simulink model "os_polyphase_synth_4096c"  (R2021a) (continued)
== system os_polyphase_synth_4096c/pfb_fir/pol1_in1_tap3 == (continued)
      | P-(P+CARRYIN)
      | P-(P+CONCAT)
      | P-(P+CONCAT+C)
      | P-(P+CONCAT+C+CARRYIN)
      | P-(P+CONCAT+CARRYIN)
      | P-(P+D)
      | P-(P+D*B)
      | P-(P+D*B+CARRYIN)
      | P-(P+D*BCIN)
      | P-(P+D*BCIN+CARRYIN)
      | P-(P+D*D)
      | P-(P+D*D+CARRYIN)
      | P-(P+D+CARRYIN)
      | P-(P+P)
      | P-(P+P+C)
      | P-(P+P+C+CARRYIN)
      | P-(P+P+CARRYIN)
      | P-A
      | P-A*A
      | P-A*A-C
      | P-A*A-C-CARRYIN
      | P-A*A-CARRYIN
      | P-A*A-P
      | P-A*A-P-CARRYIN
      | P-A*B
      | P-A*B-C
      | P-A*B-C-CARRYIN
      | P-A*B-CARRYIN
      | P-A*B-P
      | P-A*B-P-CARRYIN
      | P-A*BCIN
      | P-A*BCIN-C
      | P-A*BCIN-C-CARRYIN
      | P-A*BCIN-CARRYIN
      | P-A*BCIN-P
      | P-A*BCIN-P-CARRYIN
      | P-A-C
      | P-A-C-CARRYIN
      | P-A-CARRYIN
      | P-A-P
      | P-A-P-CARRYIN
      | P-ACIN
      | P-ACIN*ACIN
      | P-ACIN*ACIN-C
      | P-ACIN*ACIN-C-CARRYIN
      | P-ACIN*ACIN-CARRYIN
      | P-ACIN*ACIN-P
      | P-ACIN*ACIN-P-CARRYIN
      | P-ACIN*B
      | P-ACIN*B-C
      | P-ACIN*B-C-CARRYIN
      | P-ACIN*B-CARRYIN
      | P-ACIN*B-P
      | P-ACIN*B-P-CARRYIN
      | P-ACIN*BCIN
      | P-ACIN*BCIN-C
      | P-ACIN*BCIN-C-CARRYIN
      | P-ACIN*BCIN-CARRYIN
      | P-ACIN*BCIN-P
      | P-ACIN*BCIN-P-CARRYIN
      | P-ACIN-C
      | P-ACIN-C-CARRYIN
      | P-ACIN-CARRYIN
      | P-ACIN-P
      | P-ACIN-P-CARRYIN
      | P-B*(A+D)
      | P-B*(A+D)-C
      | P-B*(A+D)-C-CARRYIN
      | P-B*(A+D)-CARRYIN
      | P-B*(A+D)-P
      | P-B*(A+D)-P-CARRYIN
      | P-B*(ACIN+D)
      | P-B*(ACIN+D)-C
      | P-B*(ACIN+D)-C-CARRYIN
      | P-B*(ACIN+D)-CARRYIN
      | P-B*(ACIN+D)-P
      | P-B*(ACIN+D)-P-CARRYIN
      | P-B*(D+A)
      | P-B*(D+A)-C
      | P-B*(D+A)-C-CARRYIN
      | P-B*(D+A)-CARRYIN
      | P-B*(D+A)-P
      | P-B*(D+A)-P-CARRYIN
      | P-B*(D+ACIN)
      | P-B*(D+ACIN)-C
      | P-B*(D+ACIN)-C-CARRYIN
      | P-B*(D+ACIN)-CARRYIN
      | P-B*(D+ACIN)-P
      | P-B*(D+ACIN)-P-CARRYIN
      | P-B*(D-A)
      | P-B*(D-A)-C
      | P-B*(D-A)-C-CARRYIN
      | P-B*(D-A)-CARRYIN
      | P-B*(D-A)-P
      | P-B*(D-A)-P-CARRYIN
      | P-B*(D-ACIN)
      | P-B*(D-ACIN)-C
      | P-B*(D-ACIN)-C-CARRYIN
      | P-B*(D-ACIN)-CARRYIN
      | P-B*(D-ACIN)-P
      | P-B*(D-ACIN)-P-CARRYIN
      | P-B*A
      | P-B*A-C
      | P-B*A-C-CARRYIN
      | P-B*A-CARRYIN
      | P-B*A-P
      | P-B*A-P-CARRYIN
      | P-B*ACIN
      | P-B*ACIN-C
      | P-B*ACIN-C-CARRYIN
      | P-B*ACIN-CARRYIN
      | P-B*ACIN-P
      | P-B*ACIN-P-CARRYIN
      | P-B*D
      | P-B*D-C
      | P-B*D-C-CARRYIN
      | P-B*D-CARRYIN
      | P-B*D-P
      | P-B*D-P-CARRYIN
      | P-BCIN*(A+D)
      | P-BCIN*(A+D)-C
      | P-BCIN*(A+D)-C-CARRYIN
      | P-BCIN*(A+D)-CARRYIN
      | P-BCIN*(A+D)-P
      | P-BCIN*(A+D)-P-CARRYIN
      | P-BCIN*(ACIN+D)
      | P-BCIN*(ACIN+D)-C
      | P-BCIN*(ACIN+D)-C-CARRYIN
      | P-BCIN*(ACIN+D)-CARRYIN
      | P-BCIN*(ACIN+D)-P
      | P-BCIN*(ACIN+D)-P-CARRYIN
      | P-BCIN*(D+A)
      | P-BCIN*(D+A)-C
      | P-BCIN*(D+A)-C-CARRYIN
      | P-BCIN*(D+A)-CARRYIN
      | P-BCIN*(D+A)-P
      | P-BCIN*(D+A)-P-CARRYIN
      | P-BCIN*(D+ACIN)
      | P-BCIN*(D+ACIN)-C
      | P-BCIN*(D+ACIN)-C-CARRYIN
      | P-BCIN*(D+ACIN)-CARRYIN
      | P-BCIN*(D+ACIN)-P
      | P-BCIN*(D+ACIN)-P-CARRYIN
      | P-BCIN*(D-A)
      | P-BCIN*(D-A)-C
      | P-BCIN*(D-A)-C-CARRYIN
      | P-BCIN*(D-A)-CARRYIN
      | P-BCIN*(D-A)-P
      | P-BCIN*(D-A)-P-CARRYIN
      | P-BCIN*(D-ACIN)
      | P-BCIN*(D-ACIN)-C
      | P-BCIN*(D-ACIN)-C-CARRYIN
      | P-BCIN*(D-ACIN)-CARRYIN
      | P-BCIN*(D-ACIN)-P
      | P-BCIN*(D-ACIN)-P-CARRYIN
      | P-BCIN*A
      | P-BCIN*A-C
      | P-BCIN*A-C-CARRYIN
      | P-BCIN*A-CARRYIN
      | P-BCIN*A-P
      | P-BCIN*A-P-CARRYIN
      | P-BCIN*ACIN
      | P-BCIN*ACIN-C
      | P-BCIN*ACIN-C-CARRYIN
      | P-BCIN*ACIN-CARRYIN
      | P-BCIN*ACIN-P
      | P-BCIN*ACIN-P-CARRYIN
      | P-BCIN*D
      | P-BCIN*D-C
      | P-BCIN*D-C-CARRYIN
      | P-BCIN*D-CARRYIN
      | P-BCIN*D-P
      | P-BCIN*D-P-CARRYIN
      | P-C
      | P-C-(A+D)
      | P-C-(A+D)*(A+D)
      | P-C-(A+D)*(A+D)-CARRYIN
      | P-C-(A+D)*B
      | P-C-(A+D)*B-CARRYIN
      | P-C-(A+D)*BCIN
      | P-C-(A+D)*BCIN-CARRYIN
      | P-C-(A+D)-CARRYIN
      | P-C-(ACIN+D)
      | P-C-(ACIN+D)*(ACIN+D)
      | P-C-(ACIN+D)*(ACIN+D)-CARRYIN
      | P-C-(ACIN+D)*B
      | P-C-(ACIN+D)*B-CARRYIN
      | P-C-(ACIN+D)*BCIN
      | P-C-(ACIN+D)*BCIN-CARRYIN
      | P-C-(ACIN+D)-CARRYIN
      | P-C-(D+A)
      | P-C-(D+A)*(D+A)
      | P-C-(D+A)*(D+A)-CARRYIN
      | P-C-(D+A)*B
      | P-C-(D+A)*B-CARRYIN
      | P-C-(D+A)*BCIN
      | P-C-(D+A)*BCIN-CARRYIN
      | P-C-(D+A)-CARRYIN
      | P-C-(D+ACIN)
      | P-C-(D+ACIN)*(D+ACIN)
      | P-C-(D+ACIN)*(D+ACIN)-CARRYIN
      | P-C-(D+ACIN)*B
      | P-C-(D+ACIN)*B-CARRYIN
      | P-C-(D+ACIN)*BCIN
      | P-C-(D+ACIN)*BCIN-CARRYIN
      | P-C-(D+ACIN)-CARRYIN
      | P-C-(D-A)
      | P-C-(D-A)*(D-A)
      | P-C-(D-A)*(D-A)-CARRYIN
      | P-C-(D-A)*B
      | P-C-(D-A)*B-CARRYIN
      | P-C-(D-A)*BCIN
      | P-C-(D-A)*BCIN-CARRYIN
      | P-C-(D-A)-CARRYIN
      | P-C-(D-ACIN)
      | P-C-(D-ACIN)*(D-ACIN)
      | P-C-(D-ACIN)*(D-ACIN)-CARRYIN
      | P-C-(D-ACIN)*B
      | P-C-(D-ACIN)*B-CARRYIN
      | P-C-(D-ACIN)*BCIN
      | P-C-(D-ACIN)*BCIN-CARRYIN
      | P-C-(D-ACIN)-CARRYIN
      | P-C-A
      | P-C-A*A
      | P-C-A*A-CARRYIN
      | P-C-A*B
      | P-C-A*B-CARRYIN
      | P-C-A*BCIN
      | P-C-A*BCIN-CARRYIN
      | P-C-A-CARRYIN
      | P-C-ACIN
      | P-C-ACIN*ACIN
      | P-C-ACIN*ACIN-CARRYIN
      | P-C-ACIN*B
      | P-C-ACIN*B-CARRYIN
      | P-C-ACIN*BCIN
      | P-C-ACIN*BCIN-CARRYIN
      | P-C-ACIN-CARRYIN
      | P-C-B*(A+D)
      | P-C-B*(A+D)-CARRYIN
      | P-C-B*(ACIN+D)
      | P-C-B*(ACIN+D)-CARRYIN
      | P-C-B*(D+A)
      | P-C-B*(D+A)-CARRYIN
      | P-C-B*(D+ACIN)
      | P-C-B*(D+ACIN)-CARRYIN
      | P-C-B*(D-A)
      | P-C-B*(D-A)-CARRYIN
      | P-C-B*(D-ACIN)
      | P-C-B*(D-ACIN)-CARRYIN
      | P-C-B*A
      | P-C-B*A-CARRYIN
      | P-C-B*ACIN
      | P-C-B*ACIN-CARRYIN
      | P-C-B*D
      | P-C-B*D-CARRYIN
      | P-C-BCIN*(A+D)
      | P-C-BCIN*(A+D)-CARRYIN
      | P-C-BCIN*(ACIN+D)
      | P-C-BCIN*(ACIN+D)-CARRYIN
      | P-C-BCIN*(D+A)
      | P-C-BCIN*(D+A)-CARRYIN
      | P-C-BCIN*(D+ACIN)
      | P-C-BCIN*(D+ACIN)-CARRYIN
      | P-C-BCIN*(D-A)
      | P-C-BCIN*(D-A)-CARRYIN
      | P-C-BCIN*(D-ACIN)
      | P-C-BCIN*(D-ACIN)-CARRYIN
      | P-C-BCIN*A
      | P-C-BCIN*A-CARRYIN
      | P-C-BCIN*ACIN
      | P-C-BCIN*ACIN-CARRYIN
      | P-C-BCIN*D
      | P-C-BCIN*D-CARRYIN
      | P-C-C
      | P-C-C-CARRYIN
      | P-C-C-CONCAT
      | P-C-C-CONCAT-CARRYIN
      | P-C-C-P
      | P-C-C-P-CARRYIN
      | P-C-CARRYCASCIN
      | P-C-CARRYIN
      | P-C-CONCAT
      | P-C-CONCAT-C
      | P-C-CONCAT-C-CARRYIN
      | P-C-CONCAT-CARRYIN
      | P-C-CONCAT-P
      | P-C-CONCAT-P-CARRYIN
      | P-C-D
      | P-C-D*B
      | P-C-D*B-CARRYIN
      | P-C-D*BCIN
      | P-C-D*BCIN-CARRYIN
      | P-C-D*D
      | P-C-D*D-CARRYIN
      | P-C-D-CARRYIN
      | P-C-P
      | P-C-P-C
      | P-C-P-C-CARRYIN
      | P-C-P-CARRYIN
      | P-C-P-CONCAT
      | P-C-P-CONCAT-CARRYIN
      | P-C-P-P
      | P-C-P-P-CARRYIN
      | P-CARRYCASCIN
      | P-CARRYIN
      | P-CONCAT
      | P-CONCAT-C
      | P-CONCAT-C-C
      | P-CONCAT-C-C-CARRYIN
      | P-CONCAT-C-CARRYIN
      | P-CONCAT-C-P
      | P-CONCAT-C-P-CARRYIN
      | P-CONCAT-CARRYCASCIN
      | P-CONCAT-CARRYIN
      | P-CONCAT-P
      | P-CONCAT-P-C
      | P-CONCAT-P-C-CARRYIN
      | P-CONCAT-P-CARRYIN
      | P-D
      | P-D*B
      | P-D*B-C
      | P-D*B-C-CARRYIN
      | P-D*B-CARRYIN
      | P-D*B-P
      | P-D*B-P-CARRYIN
      | P-D*BCIN
      | P-D*BCIN-C
      | P-D*BCIN-C-CARRYIN
      | P-D*BCIN-CARRYIN
      | P-D*BCIN-P
      | P-D*BCIN-P-CARRYIN
      | P-D*D
      | P-D*D-C
      | P-D*D-C-CARRYIN
      | P-D*D-CARRYIN
      | P-D*D-P
      | P-D*D-P-CARRYIN
      | P-D-C
      | P-D-C-CARRYIN
      | P-D-CARRYIN
      | P-D-P
      | P-D-P-CARRYIN
      | P-P
      | P-P-(A+D)
      | P-P-(A+D)*(A+D)
      | P-P-(A+D)*(A+D)-CARRYIN
      | P-P-(A+D)*B
      | P-P-(A+D)*B-CARRYIN
      | P-P-(A+D)*BCIN
      | P-P-(A+D)*BCIN-CARRYIN
      | P-P-(A+D)-CARRYIN
      | P-P-(ACIN+D)
      | P-P-(ACIN+D)*(ACIN+D)
      | P-P-(ACIN+D)*(ACIN+D)-CARRYIN
      | P-P-(ACIN+D)*B
      | P-P-(ACIN+D)*B-CARRYIN
      | P-P-(ACIN+D)*BCIN
      | P-P-(ACIN+D)*BCIN-CARRYIN
      | P-P-(ACIN+D)-CARRYIN
      | P-P-(D+A)
      | P-P-(D+A)*(D+A)
      | P-P-(D+A)*(D+A)-CARRYIN
      | P-P-(D+A)*B
      | P-P-(D+A)*B-CARRYIN
      | P-P-(D+A)*BCIN
      | P-P-(D+A)*BCIN-CARRYIN
      | P-P-(D+A)-CARRYIN
      | P-P-(D+ACIN)
      | P-P-(D+ACIN)*(D+ACIN)
      | P-P-(D+ACIN)*(D+ACIN)-CARRYIN
      | P-P-(D+ACIN)*B
      | P-P-(D+ACIN)*B-CARRYIN
      | P-P-(D+ACIN)*BCIN
      | P-P-(D+ACIN)*BCIN-CARRYIN
      | P-P-(D+ACIN)-CARRYIN
      | P-P-(D-A)
      | P-P-(D-A)*(D-A)
      | P-P-(D-A)*(D-A)-CARRYIN
      | P-P-(D-A)*B
      | P-P-(D-A)*B-CARRYIN
      | P-P-(D-A)*BCIN
      | P-P-(D-A)*BCIN-CARRYIN
      | P-P-(D-A)-CARRYIN
      | P-P-(D-ACIN)
      | P-P-(D-ACIN)*(D-ACIN)
      | P-P-(D-ACIN)*(D-ACIN)-CARRYIN
      | P-P-(D-ACIN)*B
      | P-P-(D-ACIN)*B-CARRYIN
      | P-P-(D-ACIN)*BCIN
      | P-P-(D-ACIN)*BCIN-CARRYIN
      | P-P-(D-ACIN)-CARRYIN
      | P-P-A
      | P-P-A*A
      | P-P-A*A-CARRYIN
      | P-P-A*B
      | P-P-A*B-CARRYIN
      | P-P-A*BCIN
      | P-P-A*BCIN-CARRYIN
      | P-P-A-CARRYIN
      | P-P-ACIN
      | P-P-ACIN*ACIN
      | P-P-ACIN*ACIN-CARRYIN
      | P-P-ACIN*B
      | P-P-ACIN*B-CARRYIN
      | P-P-ACIN*BCIN
      | P-P-ACIN*BCIN-CARRYIN
      | P-P-ACIN-CARRYIN
      | P-P-B*(A+D)
      | P-P-B*(A+D)-CARRYIN
      | P-P-B*(ACIN+D)
      | P-P-B*(ACIN+D)-CARRYIN
      | P-P-B*(D+A)
      | P-P-B*(D+A)-CARRYIN
      | P-P-B*(D+ACIN)
      | P-P-B*(D+ACIN)-CARRYIN
      | P-P-B*(D-A)
      | P-P-B*(D-A)-CARRYIN
      | P-P-B*(D-ACIN)
      | P-P-B*(D-ACIN)-CARRYIN
      | P-P-B*A
      | P-P-B*A-CARRYIN
      | P-P-B*ACIN
      | P-P-B*ACIN-CARRYIN
      | P-P-B*D
      | P-P-B*D-CARRYIN
      | P-P-BCIN*(A+D)
      | P-P-BCIN*(A+D)-CARRYIN
      | P-P-BCIN*(ACIN+D)
      | P-P-BCIN*(ACIN+D)-CARRYIN
      | P-P-BCIN*(D+A)
      | P-P-BCIN*(D+A)-CARRYIN
      | P-P-BCIN*(D+ACIN)
      | P-P-BCIN*(D+ACIN)-CARRYIN
      | P-P-BCIN*(D-A)
      | P-P-BCIN*(D-A)-CARRYIN
      | P-P-BCIN*(D-ACIN)
      | P-P-BCIN*(D-ACIN)-CARRYIN
      | P-P-BCIN*A
      | P-P-BCIN*A-CARRYIN
      | P-P-BCIN*ACIN
      | P-P-BCIN*ACIN-CARRYIN
      | P-P-BCIN*D
      | P-P-BCIN*D-CARRYIN
      | P-P-C
      | P-P-C-C
      | P-P-C-C-CARRYIN
      | P-P-C-CARRYIN
      | P-P-C-CONCAT
      | P-P-C-CONCAT-CARRYIN
      | P-P-C-P
      | P-P-C-P-CARRYIN
      | P-P-CARRYCASCIN
      | P-P-CARRYIN
      | P-P-CONCAT
      | P-P-CONCAT-C
      | P-P-CONCAT-C-CARRYIN
      | P-P-CONCAT-CARRYIN
      | P-P-D
      | P-P-D*B
      | P-P-D*B-CARRYIN
      | P-P-D*BCIN
      | P-P-D*BCIN-CARRYIN
      | P-P-D*D
      | P-P-D*D-CARRYIN
      | P-P-D-CARRYIN
      | P-P-P
      | P-P-P-C
      | P-P-P-C-CARRYIN
      | P-P-P-CARRYIN
      | P-P>>17
      | P-PCIN
      | P-PCIN>>17
      | P>>17
      | P>>17+(A+D)
      | P>>17+(A+D)*(A+D)
      | P>>17+(A+D)*(A+D)+C
      | P>>17+(A+D)*(A+D)+C+CARRYIN
      | P>>17+(A+D)*(A+D)+CARRYIN
      | P>>17+(A+D)*(A+D)+P
      | P>>17+(A+D)*(A+D)+P+CARRYIN
      | P>>17+(A+D)*B
      | P>>17+(A+D)*B+C
      | P>>17+(A+D)*B+C+CARRYIN
      | P>>17+(A+D)*B+CARRYIN
      | P>>17+(A+D)*B+P
      | P>>17+(A+D)*B+P+CARRYIN
      | P>>17+(A+D)*BCIN
      | P>>17+(A+D)*BCIN+C
      | P>>17+(A+D)*BCIN+C+CARRYIN
      | P>>17+(A+D)*BCIN+CARRYIN
      | P>>17+(A+D)*BCIN+P
      | P>>17+(A+D)*BCIN+P+CARRYIN
      | P>>17+(A+D)+C
      | P>>17+(A+D)+C+CARRYIN
      | P>>17+(A+D)+CARRYIN
      | P>>17+(A+D)+P
      | P>>17+(A+D)+P+CARRYIN
      | P>>17+(ACIN+D)
      | P>>17+(ACIN+D)*(ACIN+D)
      | P>>17+(ACIN+D)*(ACIN+D)+C
      | P>>17+(ACIN+D)*(ACIN+D)+C+CARRYIN
      | P>>17+(ACIN+D)*(ACIN+D)+CARRYIN
      | P>>17+(ACIN+D)*(ACIN+D)+P
      | P>>17+(ACIN+D)*(ACIN+D)+P+CARRYIN
      | P>>17+(ACIN+D)*B
      | P>>17+(ACIN+D)*B+C
      | P>>17+(ACIN+D)*B+C+CARRYIN
      | P>>17+(ACIN+D)*B+CARRYIN
      | P>>17+(ACIN+D)*B+P
      | P>>17+(ACIN+D)*B+P+CARRYIN
      | P>>17+(ACIN+D)*BCIN
      | P>>17+(ACIN+D)*BCIN+C
      | P>>17+(ACIN+D)*BCIN+C+CARRYIN
      | P>>17+(ACIN+D)*BCIN+CARRYIN
      | P>>17+(ACIN+D)*BCIN+P
      | P>>17+(ACIN+D)*BCIN+P+CARRYIN
      | P>>17+(ACIN+D)+C
      | P>>17+(ACIN+D)+C+CARRYIN
      | P>>17+(ACIN+D)+CARRYIN
      | P>>17+(ACIN+D)+P
      | P>>17+(ACIN+D)+P+CARRYIN
      | P>>17+(D+A)
      | P>>17+(D+A)*(D+A)
      | P>>17+(D+A)*(D+A)+C
      | P>>17+(D+A)*(D+A)+C+CARRYIN
      | P>>17+(D+A)*(D+A)+CARRYIN
      | P>>17+(D+A)*(D+A)+P
      | P>>17+(D+A)*(D+A)+P+CARRYIN
      | P>>17+(D+A)*B
      | P>>17+(D+A)*B+C
      | P>>17+(D+A)*B+C+CARRYIN
      | P>>17+(D+A)*B+CARRYIN
      | P>>17+(D+A)*B+P
      | P>>17+(D+A)*B+P+CARRYIN
      | P>>17+(D+A)*BCIN
      | P>>17+(D+A)*BCIN+C
      | P>>17+(D+A)*BCIN+C+CARRYIN
      | P>>17+(D+A)*BCIN+CARRYIN
      | P>>17+(D+A)*BCIN+P
      | P>>17+(D+A)*BCIN+P+CARRYIN
      | P>>17+(D+A)+C
      | P>>17+(D+A)+C+CARRYIN
      | P>>17+(D+A)+CARRYIN
      | P>>17+(D+A)+P
      | P>>17+(D+A)+P+CARRYIN
      | P>>17+(D+ACIN)
      | P>>17+(D+ACIN)*(D+ACIN)
      | P>>17+(D+ACIN)*(D+ACIN)+C
      | P>>17+(D+ACIN)*(D+ACIN)+C+CARRYIN
      | P>>17+(D+ACIN)*(D+ACIN)+CARRYIN
      | P>>17+(D+ACIN)*(D+ACIN)+P
      | P>>17+(D+ACIN)*(D+ACIN)+P+CARRYIN
      | P>>17+(D+ACIN)*B
      | P>>17+(D+ACIN)*B+C
      | P>>17+(D+ACIN)*B+C+CARRYIN
      | P>>17+(D+ACIN)*B+CARRYIN
      | P>>17+(D+ACIN)*B+P
      | P>>17+(D+ACIN)*B+P+CARRYIN
      | P>>17+(D+ACIN)*BCIN
      | P>>17+(D+ACIN)*BCIN+C
      | P>>17+(D+ACIN)*BCIN+C+CARRYIN
      | P>>17+(D+ACIN)*BCIN+CARRYIN
      | P>>17+(D+ACIN)*BCIN+P
      | P>>17+(D+ACIN)*BCIN+P+CARRYIN
      | P>>17+(D+ACIN)+C
      | P>>17+(D+ACIN)+C+CARRYIN
      | P>>17+(D+ACIN)+CARRYIN
      | P>>17+(D+ACIN)+P
      | P>>17+(D+ACIN)+P+CARRYIN
      | P>>17+(D-A)
      | P>>17+(D-A)*(D-A)
      | P>>17+(D-A)*(D-A)+C
      | P>>17+(D-A)*(D-A)+C+CARRYIN
      | P>>17+(D-A)*(D-A)+CARRYIN
      | P>>17+(D-A)*(D-A)+P
      | P>>17+(D-A)*(D-A)+P+CARRYIN
      | P>>17+(D-A)*B
      | P>>17+(D-A)*B+C
      | P>>17+(D-A)*B+C+CARRYIN
      | P>>17+(D-A)*B+CARRYIN
      | P>>17+(D-A)*B+P
      | P>>17+(D-A)*B+P+CARRYIN
      | P>>17+(D-A)*BCIN
      | P>>17+(D-A)*BCIN+C
      | P>>17+(D-A)*BCIN+C+CARRYIN
      | P>>17+(D-A)*BCIN+CARRYIN
      | P>>17+(D-A)*BCIN+P
      | P>>17+(D-A)*BCIN+P+CARRYIN
      | P>>17+(D-A)+C
      | P>>17+(D-A)+C+CARRYIN
      | P>>17+(D-A)+CARRYIN
      | P>>17+(D-A)+P
      | P>>17+(D-A)+P+CARRYIN
      | P>>17+(D-ACIN)
      | P>>17+(D-ACIN)*(D-ACIN)
      | P>>17+(D-ACIN)*(D-ACIN)+C
      | P>>17+(D-ACIN)*(D-ACIN)+C+CARRYIN
      | P>>17+(D-ACIN)*(D-ACIN)+CARRYIN
      | P>>17+(D-ACIN)*(D-ACIN)+P
      | P>>17+(D-ACIN)*(D-ACIN)+P+CARRYIN
      | P>>17+(D-ACIN)*B
      | P>>17+(D-ACIN)*B+C
      | P>>17+(D-ACIN)*B+C+CARRYIN
      | P>>17+(D-ACIN)*B+CARRYIN
      | P>>17+(D-ACIN)*B+P
      | P>>17+(D-ACIN)*B+P+CARRYIN
      | P>>17+(D-ACIN)*BCIN
      | P>>17+(D-ACIN)*BCIN+C
      | P>>17+(D-ACIN)*BCIN+C+CARRYIN
      | P>>17+(D-ACIN)*BCIN+CARRYIN
      | P>>17+(D-ACIN)*BCIN+P
      | P>>17+(D-ACIN)*BCIN+P+CARRYIN
      | P>>17+(D-ACIN)+C
      | P>>17+(D-ACIN)+C+CARRYIN
      | P>>17+(D-ACIN)+CARRYIN
      | P>>17+(D-ACIN)+P
      | P>>17+(D-ACIN)+P+CARRYIN
      | P>>17+A
      | P>>17+A*A
      | P>>17+A*A+C
      | P>>17+A*A+C+CARRYIN
      | P>>17+A*A+CARRYIN
      | P>>17+A*A+P
      | P>>17+A*A+P+CARRYIN
      | P>>17+A*B
      | P>>17+A*B+C
      | P>>17+A*B+C+CARRYIN
      | P>>17+A*B+CARRYIN
      | P>>17+A*B+P
      | P>>17+A*B+P+CARRYIN
      | P>>17+A*BCIN
      | P>>17+A*BCIN+C
      | P>>17+A*BCIN+C+CARRYIN
      | P>>17+A*BCIN+CARRYIN
      | P>>17+A*BCIN+P
      | P>>17+A*BCIN+P+CARRYIN
      | P>>17+A+C
      | P>>17+A+C+CARRYIN
      | P>>17+A+CARRYIN
      | P>>17+A+P
      | P>>17+A+P+CARRYIN
      | P>>17+ACIN
      | P>>17+ACIN*ACIN
      | P>>17+ACIN*ACIN+C
      | P>>17+ACIN*ACIN+C+CARRYIN
      | P>>17+ACIN*ACIN+CARRYIN
      | P>>17+ACIN*ACIN+P
      | P>>17+ACIN*ACIN+P+CARRYIN
      | P>>17+ACIN*B
      | P>>17+ACIN*B+C
      | P>>17+ACIN*B+C+CARRYIN
      | P>>17+ACIN*B+CARRYIN
      | P>>17+ACIN*B+P
      | P>>17+ACIN*B+P+CARRYIN
      | P>>17+ACIN*BCIN
      | P>>17+ACIN*BCIN+C
      | P>>17+ACIN*BCIN+C+CARRYIN
      | P>>17+ACIN*BCIN+CARRYIN
      | P>>17+ACIN*BCIN+P
      | P>>17+ACIN*BCIN+P+CARRYIN
      | P>>17+ACIN+C
      | P>>17+ACIN+C+CARRYIN
      | P>>17+ACIN+CARRYIN
      | P>>17+ACIN+P
      | P>>17+ACIN+P+CARRYIN
      | P>>17+B*(A+D)
      | P>>17+B*(A+D)+C
      | P>>17+B*(A+D)+C+CARRYIN
      | P>>17+B*(A+D)+CARRYIN
      | P>>17+B*(A+D)+P
      | P>>17+B*(A+D)+P+CARRYIN
      | P>>17+B*(ACIN+D)
      | P>>17+B*(ACIN+D)+C
      | P>>17+B*(ACIN+D)+C+CARRYIN
      | P>>17+B*(ACIN+D)+CARRYIN
      | P>>17+B*(ACIN+D)+P
      | P>>17+B*(ACIN+D)+P+CARRYIN
      | P>>17+B*(D+A)
      | P>>17+B*(D+A)+C
      | P>>17+B*(D+A)+C+CARRYIN
      | P>>17+B*(D+A)+CARRYIN
      | P>>17+B*(D+A)+P
      | P>>17+B*(D+A)+P+CARRYIN
      | P>>17+B*(D+ACIN)
      | P>>17+B*(D+ACIN)+C
      | P>>17+B*(D+ACIN)+C+CARRYIN
      | P>>17+B*(D+ACIN)+CARRYIN
      | P>>17+B*(D+ACIN)+P
      | P>>17+B*(D+ACIN)+P+CARRYIN
      | P>>17+B*(D-A)
      | P>>17+B*(D-A)+C
      | P>>17+B*(D-A)+C+CARRYIN
      | P>>17+B*(D-A)+CARRYIN
      | P>>17+B*(D-A)+P
      | P>>17+B*(D-A)+P+CARRYIN
      | P>>17+B*(D-ACIN)
      | P>>17+B*(D-ACIN)+C
      | P>>17+B*(D-ACIN)+C+CARRYIN
      | P>>17+B*(D-ACIN)+CARRYIN
      | P>>17+B*(D-ACIN)+P
      | P>>17+B*(D-ACIN)+P+CARRYIN
      | P>>17+B*A
      | P>>17+B*A+C
      | P>>17+B*A+C+CARRYIN
      | P>>17+B*A+CARRYIN
      | P>>17+B*A+P
      | P>>17+B*A+P+CARRYIN
      | P>>17+B*ACIN
      | P>>17+B*ACIN+C
      | P>>17+B*ACIN+C+CARRYIN
      | P>>17+B*ACIN+CARRYIN
      | P>>17+B*ACIN+P
      | P>>17+B*ACIN+P+CARRYIN
      | P>>17+B*D
      | P>>17+B*D+C
      | P>>17+B*D+C+CARRYIN
      | P>>17+B*D+CARRYIN
      | P>>17+B*D+P
      | P>>17+B*D+P+CARRYIN
      | P>>17+BCIN*(A+D)
      | P>>17+BCIN*(A+D)+C
      | P>>17+BCIN*(A+D)+C+CARRYIN
      | P>>17+BCIN*(A+D)+CARRYIN
      | P>>17+BCIN*(A+D)+P
      | P>>17+BCIN*(A+D)+P+CARRYIN
      | P>>17+BCIN*(ACIN+D)
      | P>>17+BCIN*(ACIN+D)+C
      | P>>17+BCIN*(ACIN+D)+C+CARRYIN
      | P>>17+BCIN*(ACIN+D)+CARRYIN
      | P>>17+BCIN*(ACIN+D)+P
      | P>>17+BCIN*(ACIN+D)+P+CARRYIN
      | P>>17+BCIN*(D+A)
      | P>>17+BCIN*(D+A)+C
      | P>>17+BCIN*(D+A)+C+CARRYIN
      | P>>17+BCIN*(D+A)+CARRYIN
      | P>>17+BCIN*(D+A)+P
      | P>>17+BCIN*(D+A)+P+CARRYIN
      | P>>17+BCIN*(D+ACIN)
      | P>>17+BCIN*(D+ACIN)+C
      | P>>17+BCIN*(D+ACIN)+C+CARRYIN
      | P>>17+BCIN*(D+ACIN)+CARRYIN
      | P>>17+BCIN*(D+ACIN)+P
      | P>>17+BCIN*(D+ACIN)+P+CARRYIN
      | P>>17+BCIN*(D-A)
      | P>>17+BCIN*(D-A)+C
      | P>>17+BCIN*(D-A)+C+CARRYIN
      | P>>17+BCIN*(D-A)+CARRYIN
      | P>>17+BCIN*(D-A)+P
      | P>>17+BCIN*(D-A)+P+CARRYIN
      | P>>17+BCIN*(D-ACIN)
      | P>>17+BCIN*(D-ACIN)+C
      | P>>17+BCIN*(D-ACIN)+C+CARRYIN
      | P>>17+BCIN*(D-ACIN)+CARRYIN
      | P>>17+BCIN*(D-ACIN)+P
      | P>>17+BCIN*(D-ACIN)+P+CARRYIN
      | P>>17+BCIN*A
      | P>>17+BCIN*A+C
      | P>>17+BCIN*A+C+CARRYIN
      | P>>17+BCIN*A+CARRYIN
      | P>>17+BCIN*A+P
      | P>>17+BCIN*A+P+CARRYIN
      | P>>17+BCIN*ACIN
      | P>>17+BCIN*ACIN+C
      | P>>17+BCIN*ACIN+C+CARRYIN
      | P>>17+BCIN*ACIN+CARRYIN
      | P>>17+BCIN*ACIN+P
      | P>>17+BCIN*ACIN+P+CARRYIN
      | P>>17+BCIN*D
      | P>>17+BCIN*D+C
      | P>>17+BCIN*D+C+CARRYIN
      | P>>17+BCIN*D+CARRYIN
      | P>>17+BCIN*D+P
      | P>>17+BCIN*D+P+CARRYIN
      | P>>17+C
      | P>>17+C+(A+D)
      | P>>17+C+(A+D)*(A+D)
      | P>>17+C+(A+D)*(A+D)+CARRYIN
      | P>>17+C+(A+D)*B
      | P>>17+C+(A+D)*B+CARRYIN
      | P>>17+C+(A+D)*BCIN
      | P>>17+C+(A+D)*BCIN+CARRYIN
      | P>>17+C+(A+D)+CARRYIN
      | P>>17+C+(ACIN+D)
      | P>>17+C+(ACIN+D)*(ACIN+D)
      | P>>17+C+(ACIN+D)*(ACIN+D)+CARRYIN
      | P>>17+C+(ACIN+D)*B
      | P>>17+C+(ACIN+D)*B+CARRYIN
      | P>>17+C+(ACIN+D)*BCIN
      | P>>17+C+(ACIN+D)*BCIN+CARRYIN
      | P>>17+C+(ACIN+D)+CARRYIN
      | P>>17+C+(D+A)
      | P>>17+C+(D+A)*(D+A)
      | P>>17+C+(D+A)*(D+A)+CARRYIN
      | P>>17+C+(D+A)*B
      | P>>17+C+(D+A)*B+CARRYIN
      | P>>17+C+(D+A)*BCIN
      | P>>17+C+(D+A)*BCIN+CARRYIN
      | P>>17+C+(D+A)+CARRYIN
      | P>>17+C+(D+ACIN)
      | P>>17+C+(D+ACIN)*(D+ACIN)
      | P>>17+C+(D+ACIN)*(D+ACIN)+CARRYIN
      | P>>17+C+(D+ACIN)*B
      | P>>17+C+(D+ACIN)*B+CARRYIN
      | P>>17+C+(D+ACIN)*BCIN
      | P>>17+C+(D+ACIN)*BCIN+CARRYIN
      | P>>17+C+(D+ACIN)+CARRYIN
      | P>>17+C+(D-A)
      | P>>17+C+(D-A)*(D-A)
      | P>>17+C+(D-A)*(D-A)+CARRYIN
      | P>>17+C+(D-A)*B
      | P>>17+C+(D-A)*B+CARRYIN
      | P>>17+C+(D-A)*BCIN
      | P>>17+C+(D-A)*BCIN+CARRYIN
      | P>>17+C+(D-A)+CARRYIN
      | P>>17+C+(D-ACIN)
      | P>>17+C+(D-ACIN)*(D-ACIN)
      | P>>17+C+(D-ACIN)*(D-ACIN)+CARRYIN
      | P>>17+C+(D-ACIN)*B
      | P>>17+C+(D-ACIN)*B+CARRYIN
      | P>>17+C+(D-ACIN)*BCIN
      | P>>17+C+(D-ACIN)*BCIN+CARRYIN
      | P>>17+C+(D-ACIN)+CARRYIN
      | P>>17+C+A
      | P>>17+C+A*A
      | P>>17+C+A*A+CARRYIN
      | P>>17+C+A*B
      | P>>17+C+A*B+CARRYIN
      | P>>17+C+A*BCIN
      | P>>17+C+A*BCIN+CARRYIN
      | P>>17+C+A+CARRYIN
      | P>>17+C+ACIN
      | P>>17+C+ACIN*ACIN
      | P>>17+C+ACIN*ACIN+CARRYIN
      | P>>17+C+ACIN*B
      | P>>17+C+ACIN*B+CARRYIN
      | P>>17+C+ACIN*BCIN
      | P>>17+C+ACIN*BCIN+CARRYIN
      | P>>17+C+ACIN+CARRYIN
      | P>>17+C+B*(A+D)
      | P>>17+C+B*(A+D)+CARRYIN
      | P>>17+C+B*(ACIN+D)
      | P>>17+C+B*(ACIN+D)+CARRYIN
      | P>>17+C+B*(D+A)
      | P>>17+C+B*(D+A)+CARRYIN
      | P>>17+C+B*(D+ACIN)
      | P>>17+C+B*(D+ACIN)+CARRYIN
      | P>>17+C+B*(D-A)
      | P>>17+C+B*(D-A)+CARRYIN
      | P>>17+C+B*(D-ACIN)
      | P>>17+C+B*(D-ACIN)+CARRYIN
      | P>>17+C+B*A
      | P>>17+C+B*A+CARRYIN
      | P>>17+C+B*ACIN
      | P>>17+C+B*ACIN+CARRYIN
      | P>>17+C+B*D
      | P>>17+C+B*D+CARRYIN
      | P>>17+C+BCIN*(A+D)
      | P>>17+C+BCIN*(A+D)+CARRYIN
      | P>>17+C+BCIN*(ACIN+D)
      | P>>17+C+BCIN*(ACIN+D)+CARRYIN
      | P>>17+C+BCIN*(D+A)
      | P>>17+C+BCIN*(D+A)+CARRYIN
      | P>>17+C+BCIN*(D+ACIN)
      | P>>17+C+BCIN*(D+ACIN)+CARRYIN
      | P>>17+C+BCIN*(D-A)
      | P>>17+C+BCIN*(D-A)+CARRYIN
      | P>>17+C+BCIN*(D-ACIN)
      | P>>17+C+BCIN*(D-ACIN)+CARRYIN
      | P>>17+C+BCIN*A
      | P>>17+C+BCIN*A+CARRYIN
      | P>>17+C+BCIN*ACIN
      | P>>17+C+BCIN*ACIN+CARRYIN
      | P>>17+C+BCIN*D
      | P>>17+C+BCIN*D+CARRYIN
      | P>>17+C+C
      | P>>17+C+C+CARRYIN
      | P>>17+C+C+CONCAT
      | P>>17+C+C+CONCAT+CARRYIN
      | P>>17+C+C+P
      | P>>17+C+C+P+CARRYIN
      | P>>17+C+CARRYIN
      | P>>17+C+CONCAT
      | P>>17+C+CONCAT+C
      | P>>17+C+CONCAT+C+CARRYIN
      | P>>17+C+CONCAT+CARRYIN
      | P>>17+C+CONCAT+P
      | P>>17+C+CONCAT+P+CARRYIN
      | P>>17+C+D
      | P>>17+C+D*B
      | P>>17+C+D*B+CARRYIN
      | P>>17+C+D*BCIN
      | P>>17+C+D*BCIN+CARRYIN
      | P>>17+C+D*D
      | P>>17+C+D*D+CARRYIN
      | P>>17+C+D+CARRYIN
      | P>>17+C+P
      | P>>17+C+P+C
      | P>>17+C+P+C+CARRYIN
      | P>>17+C+P+CARRYIN
      | P>>17+C+P+CONCAT
      | P>>17+C+P+CONCAT+CARRYIN
      | P>>17+C+P+P
      | P>>17+C+P+P+CARRYIN
      | P>>17+CARRYIN
      | P>>17+CONCAT
      | P>>17+CONCAT+C
      | P>>17+CONCAT+C+C
      | P>>17+CONCAT+C+C+CARRYIN
      | P>>17+CONCAT+C+CARRYIN
      | P>>17+CONCAT+C+P
      | P>>17+CONCAT+C+P+CARRYIN
      | P>>17+CONCAT+CARRYIN
      | P>>17+CONCAT+P
      | P>>17+CONCAT+P+C
      | P>>17+CONCAT+P+C+CARRYIN
      | P>>17+CONCAT+P+CARRYIN
      | P>>17+D
      | P>>17+D*B
      | P>>17+D*B+C
      | P>>17+D*B+C+CARRYIN
      | P>>17+D*B+CARRYIN
      | P>>17+D*B+P
      | P>>17+D*B+P+CARRYIN
      | P>>17+D*BCIN
      | P>>17+D*BCIN+C
      | P>>17+D*BCIN+C+CARRYIN
      | P>>17+D*BCIN+CARRYIN
      | P>>17+D*BCIN+P
      | P>>17+D*BCIN+P+CARRYIN
      | P>>17+D*D
      | P>>17+D*D+C
      | P>>17+D*D+C+CARRYIN
      | P>>17+D*D+CARRYIN
      | P>>17+D*D+P
      | P>>17+D*D+P+CARRYIN
      | P>>17+D+C
      | P>>17+D+C+CARRYIN
      | P>>17+D+CARRYIN
      | P>>17+D+P
      | P>>17+D+P+CARRYIN
      | P>>17+P
      | P>>17+P+(A+D)
      | P>>17+P+(A+D)*(A+D)
      | P>>17+P+(A+D)*(A+D)+CARRYIN
      | P>>17+P+(A+D)*B
      | P>>17+P+(A+D)*B+CARRYIN
      | P>>17+P+(A+D)*BCIN
      | P>>17+P+(A+D)*BCIN+CARRYIN
      | P>>17+P+(A+D)+CARRYIN
      | P>>17+P+(ACIN+D)
      | P>>17+P+(ACIN+D)*(ACIN+D)
      | P>>17+P+(ACIN+D)*(ACIN+D)+CARRYIN
      | P>>17+P+(ACIN+D)*B
      | P>>17+P+(ACIN+D)*B+CARRYIN
      | P>>17+P+(ACIN+D)*BCIN
      | P>>17+P+(ACIN+D)*BCIN+CARRYIN
      | P>>17+P+(ACIN+D)+CARRYIN
      | P>>17+P+(D+A)
      | P>>17+P+(D+A)*(D+A)
      | P>>17+P+(D+A)*(D+A)+CARRYIN
      | P>>17+P+(D+A)*B
      | P>>17+P+(D+A)*B+CARRYIN
      | P>>17+P+(D+A)*BCIN
      | P>>17+P+(D+A)*BCIN+CARRYIN
      | P>>17+P+(D+A)+CARRYIN
      | P>>17+P+(D+ACIN)
      | P>>17+P+(D+ACIN)*(D+ACIN)
      | P>>17+P+(D+ACIN)*(D+ACIN)+CARRYIN
      | P>>17+P+(D+ACIN)*B
      | P>>17+P+(D+ACIN)*B+CARRYIN
      | P>>17+P+(D+ACIN)*BCIN
      | P>>17+P+(D+ACIN)*BCIN+CARRYIN
      | P>>17+P+(D+ACIN)+CARRYIN
      | P>>17+P+(D-A)
      | P>>17+P+(D-A)*(D-A)
      | P>>17+P+(D-A)*(D-A)+CARRYIN
      | P>>17+P+(D-A)*B
      | P>>17+P+(D-A)*B+CARRYIN
      | P>>17+P+(D-A)*BCIN
      | P>>17+P+(D-A)*BCIN+CARRYIN
      | P>>17+P+(D-A)+CARRYIN
      | P>>17+P+(D-ACIN)
      | P>>17+P+(D-ACIN)*(D-ACIN)
      | P>>17+P+(D-ACIN)*(D-ACIN)+CARRYIN
      | P>>17+P+(D-ACIN)*B
      | P>>17+P+(D-ACIN)*B+CARRYIN
      | P>>17+P+(D-ACIN)*BCIN
      | P>>17+P+(D-ACIN)*BCIN+CARRYIN
      | P>>17+P+(D-ACIN)+CARRYIN
      | P>>17+P+A
      | P>>17+P+A*A
      | P>>17+P+A*A+CARRYIN
      | P>>17+P+A*B
      | P>>17+P+A*B+CARRYIN
      | P>>17+P+A*BCIN
      | P>>17+P+A*BCIN+CARRYIN
      | P>>17+P+A+CARRYIN
      | P>>17+P+ACIN
      | P>>17+P+ACIN*ACIN
      | P>>17+P+ACIN*ACIN+CARRYIN
      | P>>17+P+ACIN*B
      | P>>17+P+ACIN*B+CARRYIN
      | P>>17+P+ACIN*BCIN
      | P>>17+P+ACIN*BCIN+CARRYIN
      | P>>17+P+ACIN+CARRYIN
      | P>>17+P+B*(A+D)
      | P>>17+P+B*(A+D)+CARRYIN
      | P>>17+P+B*(ACIN+D)
      | P>>17+P+B*(ACIN+D)+CARRYIN
      | P>>17+P+B*(D+A)
      | P>>17+P+B*(D+A)+CARRYIN
      | P>>17+P+B*(D+ACIN)
      | P>>17+P+B*(D+ACIN)+CARRYIN
      | P>>17+P+B*(D-A)
      | P>>17+P+B*(D-A)+CARRYIN
      | P>>17+P+B*(D-ACIN)
      | P>>17+P+B*(D-ACIN)+CARRYIN
      | P>>17+P+B*A
      | P>>17+P+B*A+CARRYIN
      | P>>17+P+B*ACIN
      | P>>17+P+B*ACIN+CARRYIN
      | P>>17+P+B*D
      | P>>17+P+B*D+CARRYIN
      | P>>17+P+BCIN*(A+D)
      | P>>17+P+BCIN*(A+D)+CARRYIN
      | P>>17+P+BCIN*(ACIN+D)
      | P>>17+P+BCIN*(ACIN+D)+CARRYIN
      | P>>17+P+BCIN*(D+A)
      | P>>17+P+BCIN*(D+A)+CARRYIN
      | P>>17+P+BCIN*(D+ACIN)
      | P>>17+P+BCIN*(D+ACIN)+CARRYIN
      | P>>17+P+BCIN*(D-A)
      | P>>17+P+BCIN*(D-A)+CARRYIN
      | P>>17+P+BCIN*(D-ACIN)
      | P>>17+P+BCIN*(D-ACIN)+CARRYIN
      | P>>17+P+BCIN*A
      | P>>17+P+BCIN*A+CARRYIN
      | P>>17+P+BCIN*ACIN
      | P>>17+P+BCIN*ACIN+CARRYIN
      | P>>17+P+BCIN*D
      | P>>17+P+BCIN*D+CARRYIN
      | P>>17+P+C
      | P>>17+P+C+C
      | P>>17+P+C+C+CARRYIN
      | P>>17+P+C+CARRYIN
      | P>>17+P+C+CONCAT
      | P>>17+P+C+CONCAT+CARRYIN
      | P>>17+P+C+P
      | P>>17+P+C+P+CARRYIN
      | P>>17+P+CARRYIN
      | P>>17+P+CONCAT
      | P>>17+P+CONCAT+C
      | P>>17+P+CONCAT+C+CARRYIN
      | P>>17+P+CONCAT+CARRYIN
      | P>>17+P+D
      | P>>17+P+D*B
      | P>>17+P+D*B+CARRYIN
      | P>>17+P+D*BCIN
      | P>>17+P+D*BCIN+CARRYIN
      | P>>17+P+D*D
      | P>>17+P+D*D+CARRYIN
      | P>>17+P+D+CARRYIN
      | P>>17+P+P
      | P>>17+P+P+C
      | P>>17+P+P+C+CARRYIN
      | P>>17+P+P+CARRYIN
      | P>>17-((A+D))
      | P>>17-((A+D)*(A+D))
      | P>>17-((A+D)*(A+D)+C)
      | P>>17-((A+D)*(A+D)+C+CARRYIN)
      | P>>17-((A+D)*(A+D)+CARRYIN)
      | P>>17-((A+D)*(A+D)+P)
      | P>>17-((A+D)*(A+D)+P+CARRYIN)
      | P>>17-((A+D)*B)
      | P>>17-((A+D)*B+C)
      | P>>17-((A+D)*B+C+CARRYIN)
      | P>>17-((A+D)*B+CARRYIN)
      | P>>17-((A+D)*B+P)
      | P>>17-((A+D)*B+P+CARRYIN)
      | P>>17-((A+D)*BCIN)
      | P>>17-((A+D)*BCIN+C)
      | P>>17-((A+D)*BCIN+C+CARRYIN)
      | P>>17-((A+D)*BCIN+CARRYIN)
      | P>>17-((A+D)*BCIN+P)
      | P>>17-((A+D)*BCIN+P+CARRYIN)
      | P>>17-((A+D)+C)
      | P>>17-((A+D)+C+CARRYIN)
      | P>>17-((A+D)+CARRYIN)
      | P>>17-((A+D)+P)
      | P>>17-((A+D)+P+CARRYIN)
      | P>>17-((ACIN+D))
      | P>>17-((ACIN+D)*(ACIN+D))
      | P>>17-((ACIN+D)*(ACIN+D)+C)
      | P>>17-((ACIN+D)*(ACIN+D)+C+CARRYIN)
      | P>>17-((ACIN+D)*(ACIN+D)+CARRYIN)
      | P>>17-((ACIN+D)*(ACIN+D)+P)
      | P>>17-((ACIN+D)*(ACIN+D)+P+CARRYIN)
      | P>>17-((ACIN+D)*B)
      | P>>17-((ACIN+D)*B+C)
      | P>>17-((ACIN+D)*B+C+CARRYIN)
      | P>>17-((ACIN+D)*B+CARRYIN)
      | P>>17-((ACIN+D)*B+P)
      | P>>17-((ACIN+D)*B+P+CARRYIN)
      | P>>17-((ACIN+D)*BCIN)
      | P>>17-((ACIN+D)*BCIN+C)
      | P>>17-((ACIN+D)*BCIN+C+CARRYIN)
      | P>>17-((ACIN+D)*BCIN+CARRYIN)
      | P>>17-((ACIN+D)*BCIN+P)
      | P>>17-((ACIN+D)*BCIN+P+CARRYIN)
      | P>>17-((ACIN+D)+C)
      | P>>17-((ACIN+D)+C+CARRYIN)
      | P>>17-((ACIN+D)+CARRYIN)
      | P>>17-((ACIN+D)+P)
      | P>>17-((ACIN+D)+P+CARRYIN)
      | P>>17-((D+A))
      | P>>17-((D+A)*(D+A))
      | P>>17-((D+A)*(D+A)+C)
      | P>>17-((D+A)*(D+A)+C+CARRYIN)
      | P>>17-((D+A)*(D+A)+CARRYIN)
      | P>>17-((D+A)*(D+A)+P)
      | P>>17-((D+A)*(D+A)+P+CARRYIN)
      | P>>17-((D+A)*B)
      | P>>17-((D+A)*B+C)
      | P>>17-((D+A)*B+C+CARRYIN)
      | P>>17-((D+A)*B+CARRYIN)
      | P>>17-((D+A)*B+P)
      | P>>17-((D+A)*B+P+CARRYIN)
      | P>>17-((D+A)*BCIN)
      | P>>17-((D+A)*BCIN+C)
      | P>>17-((D+A)*BCIN+C+CARRYIN)
      | P>>17-((D+A)*BCIN+CARRYIN)
      | P>>17-((D+A)*BCIN+P)
      | P>>17-((D+A)*BCIN+P+CARRYIN)
      | P>>17-((D+A)+C)
      | P>>17-((D+A)+C+CARRYIN)
      | P>>17-((D+A)+CARRYIN)
      | P>>17-((D+A)+P)
      | P>>17-((D+A)+P+CARRYIN)
      | P>>17-((D+ACIN))
      | P>>17-((D+ACIN)*(D+ACIN))
      | P>>17-((D+ACIN)*(D+ACIN)+C)
      | P>>17-((D+ACIN)*(D+ACIN)+C+CARRYIN)
      | P>>17-((D+ACIN)*(D+ACIN)+CARRYIN)
      | P>>17-((D+ACIN)*(D+ACIN)+P)
      | P>>17-((D+ACIN)*(D+ACIN)+P+CARRYIN)
      | P>>17-((D+ACIN)*B)
      | P>>17-((D+ACIN)*B+C)
      | P>>17-((D+ACIN)*B+C+CARRYIN)
      | P>>17-((D+ACIN)*B+CARRYIN)
      | P>>17-((D+ACIN)*B+P)
      | P>>17-((D+ACIN)*B+P+CARRYIN)
      | P>>17-((D+ACIN)*BCIN)
      | P>>17-((D+ACIN)*BCIN+C)
      | P>>17-((D+ACIN)*BCIN+C+CARRYIN)
      | P>>17-((D+ACIN)*BCIN+CARRYIN)
      | P>>17-((D+ACIN)*BCIN+P)
      | P>>17-((D+ACIN)*BCIN+P+CARRYIN)
      | P>>17-((D+ACIN)+C)
      | P>>17-((D+ACIN)+C+CARRYIN)
      | P>>17-((D+ACIN)+CARRYIN)
      | P>>17-((D+ACIN)+P)
      | P>>17-((D+ACIN)+P+CARRYIN)
      | P>>17-((D-A))
      | P>>17-((D-A)*(D-A))
      | P>>17-((D-A)*(D-A)+C)
      | P>>17-((D-A)*(D-A)+C+CARRYIN)
      | P>>17-((D-A)*(D-A)+CARRYIN)
      | P>>17-((D-A)*(D-A)+P)
      | P>>17-((D-A)*(D-A)+P+CARRYIN)
      | P>>17-((D-A)*B)
      | P>>17-((D-A)*B+C)
      | P>>17-((D-A)*B+C+CARRYIN)
      | P>>17-((D-A)*B+CARRYIN)
      | P>>17-((D-A)*B+P)
      | P>>17-((D-A)*B+P+CARRYIN)
      | P>>17-((D-A)*BCIN)
      | P>>17-((D-A)*BCIN+C)
      | P>>17-((D-A)*BCIN+C+CARRYIN)
      | P>>17-((D-A)*BCIN+CARRYIN)
      | P>>17-((D-A)*BCIN+P)
      | P>>17-((D-A)*BCIN+P+CARRYIN)
      | P>>17-((D-A)+C)
      | P>>17-((D-A)+C+CARRYIN)
      | P>>17-((D-A)+CARRYIN)
      | P>>17-((D-A)+P)
      | P>>17-((D-A)+P+CARRYIN)
      | P>>17-((D-ACIN))
      | P>>17-((D-ACIN)*(D-ACIN))
      | P>>17-((D-ACIN)*(D-ACIN)+C)
      | P>>17-((D-ACIN)*(D-ACIN)+C+CARRYIN)
      | P>>17-((D-ACIN)*(D-ACIN)+CARRYIN)
      | P>>17-((D-ACIN)*(D-ACIN)+P)
      | P>>17-((D-ACIN)*(D-ACIN)+P+CARRYIN)
      | P>>17-((D-ACIN)*B)
      | P>>17-((D-ACIN)*B+C)
      | P>>17-((D-ACIN)*B+C+CARRYIN)
      | P>>17-((D-ACIN)*B+CARRYIN)
      | P>>17-((D-ACIN)*B+P)
      | P>>17-((D-ACIN)*B+P+CARRYIN)
      | P>>17-((D-ACIN)*BCIN)
      | P>>17-((D-ACIN)*BCIN+C)
      | P>>17-((D-ACIN)*BCIN+C+CARRYIN)
      | P>>17-((D-ACIN)*BCIN+CARRYIN)
      | P>>17-((D-ACIN)*BCIN+P)
      | P>>17-((D-ACIN)*BCIN+P+CARRYIN)
      | P>>17-((D-ACIN)+C)
      | P>>17-((D-ACIN)+C+CARRYIN)
      | P>>17-((D-ACIN)+CARRYIN)
      | P>>17-((D-ACIN)+P)
      | P>>17-((D-ACIN)+P+CARRYIN)
      | P>>17-(A)
      | P>>17-(A*A)
      | P>>17-(A*A+C)
      | P>>17-(A*A+C+CARRYIN)
      | P>>17-(A*A+CARRYIN)
      | P>>17-(A*A+P)
      | P>>17-(A*A+P+CARRYIN)
      | P>>17-(A*B)
      | P>>17-(A*B+C)
      | P>>17-(A*B+C+CARRYIN)
      | P>>17-(A*B+CARRYIN)
      | P>>17-(A*B+P)
      | P>>17-(A*B+P+CARRYIN)
      | P>>17-(A*BCIN)
      | P>>17-(A*BCIN+C)
      | P>>17-(A*BCIN+C+CARRYIN)
      | P>>17-(A*BCIN+CARRYIN)
      | P>>17-(A*BCIN+P)
      | P>>17-(A*BCIN+P+CARRYIN)
      | P>>17-(A+C)
      | P>>17-(A+C+CARRYIN)
      | P>>17-(A+CARRYIN)
      | P>>17-(A+D)
      | P>>17-(A+D)*(A+D)
      | P>>17-(A+D)*(A+D)-C
      | P>>17-(A+D)*(A+D)-C-CARRYIN
      | P>>17-(A+D)*(A+D)-CARRYIN
      | P>>17-(A+D)*(A+D)-P
      | P>>17-(A+D)*(A+D)-P-CARRYIN
      | P>>17-(A+D)*B
      | P>>17-(A+D)*B-C
      | P>>17-(A+D)*B-C-CARRYIN
      | P>>17-(A+D)*B-CARRYIN
      | P>>17-(A+D)*B-P
      | P>>17-(A+D)*B-P-CARRYIN
      | P>>17-(A+D)*BCIN
      | P>>17-(A+D)*BCIN-C
      | P>>17-(A+D)*BCIN-C-CARRYIN
      | P>>17-(A+D)*BCIN-CARRYIN
      | P>>17-(A+D)*BCIN-P
      | P>>17-(A+D)*BCIN-P-CARRYIN
      | P>>17-(A+D)-C
      | P>>17-(A+D)-C-CARRYIN
      | P>>17-(A+D)-CARRYIN
      | P>>17-(A+D)-P
      | P>>17-(A+D)-P-CARRYIN
      | P>>17-(A+P)
      | P>>17-(A+P+CARRYIN)
      | P>>17-(ACIN)
      | P>>17-(ACIN*ACIN)
      | P>>17-(ACIN*ACIN+C)
      | P>>17-(ACIN*ACIN+C+CARRYIN)
      | P>>17-(ACIN*ACIN+CARRYIN)
      | P>>17-(ACIN*ACIN+P)
      | P>>17-(ACIN*ACIN+P+CARRYIN)
      | P>>17-(ACIN*B)
      | P>>17-(ACIN*B+C)
      | P>>17-(ACIN*B+C+CARRYIN)
      | P>>17-(ACIN*B+CARRYIN)
      | P>>17-(ACIN*B+P)
      | P>>17-(ACIN*B+P+CARRYIN)
      | P>>17-(ACIN*BCIN)
      | P>>17-(ACIN*BCIN+C)
      | P>>17-(ACIN*BCIN+C+CARRYIN)
      | P>>17-(ACIN*BCIN+CARRYIN)
      | P>>17-(ACIN*BCIN+P)
      | P>>17-(ACIN*BCIN+P+CARRYIN)
      | P>>17-(ACIN+C)
      | P>>17-(ACIN+C+CARRYIN)
      | P>>17-(ACIN+CARRYIN)
      | P>>17-(ACIN+D)
      | P>>17-(ACIN+D)*(ACIN+D)
      | P>>17-(ACIN+D)*(ACIN+D)-C
      | P>>17-(ACIN+D)*(ACIN+D)-C-CARRYIN
      | P>>17-(ACIN+D)*(ACIN+D)-CARRYIN
      | P>>17-(ACIN+D)*(ACIN+D)-P
      | P>>17-(ACIN+D)*(ACIN+D)-P-CARRYIN
      | P>>17-(ACIN+D)*B
      | P>>17-(ACIN+D)*B-C
      | P>>17-(ACIN+D)*B-C-CARRYIN
      | P>>17-(ACIN+D)*B-CARRYIN
      | P>>17-(ACIN+D)*B-P
      | P>>17-(ACIN+D)*B-P-CARRYIN
      | P>>17-(ACIN+D)*BCIN
      | P>>17-(ACIN+D)*BCIN-C
      | P>>17-(ACIN+D)*BCIN-C-CARRYIN
      | P>>17-(ACIN+D)*BCIN-CARRYIN
      | P>>17-(ACIN+D)*BCIN-P
      | P>>17-(ACIN+D)*BCIN-P-CARRYIN
      | P>>17-(ACIN+D)-C
      | P>>17-(ACIN+D)-C-CARRYIN
      | P>>17-(ACIN+D)-CARRYIN
      | P>>17-(ACIN+D)-P
      | P>>17-(ACIN+D)-P-CARRYIN
      | P>>17-(ACIN+P)
      | P>>17-(ACIN+P+CARRYIN)
      | P>>17-(B*(A+D))
      | P>>17-(B*(A+D)+C)
      | P>>17-(B*(A+D)+C+CARRYIN)
      | P>>17-(B*(A+D)+CARRYIN)
      | P>>17-(B*(A+D)+P)
      | P>>17-(B*(A+D)+P+CARRYIN)
      | P>>17-(B*(ACIN+D))
      | P>>17-(B*(ACIN+D)+C)
      | P>>17-(B*(ACIN+D)+C+CARRYIN)
      | P>>17-(B*(ACIN+D)+CARRYIN)
      | P>>17-(B*(ACIN+D)+P)
      | P>>17-(B*(ACIN+D)+P+CARRYIN)
      | P>>17-(B*(D+A))
      | P>>17-(B*(D+A)+C)
      | P>>17-(B*(D+A)+C+CARRYIN)
      | P>>17-(B*(D+A)+CARRYIN)
      | P>>17-(B*(D+A)+P)
      | P>>17-(B*(D+A)+P+CARRYIN)
      | P>>17-(B*(D+ACIN))
      | P>>17-(B*(D+ACIN)+C)
      | P>>17-(B*(D+ACIN)+C+CARRYIN)
      | P>>17-(B*(D+ACIN)+CARRYIN)
      | P>>17-(B*(D+ACIN)+P)
      | P>>17-(B*(D+ACIN)+P+CARRYIN)
      | P>>17-(B*(D-A))
      | P>>17-(B*(D-A)+C)
      | P>>17-(B*(D-A)+C+CARRYIN)
      | P>>17-(B*(D-A)+CARRYIN)
      | P>>17-(B*(D-A)+P)
      | P>>17-(B*(D-A)+P+CARRYIN)
      | P>>17-(B*(D-ACIN))
      | P>>17-(B*(D-ACIN)+C)
      | P>>17-(B*(D-ACIN)+C+CARRYIN)
      | P>>17-(B*(D-ACIN)+CARRYIN)
      | P>>17-(B*(D-ACIN)+P)
      | P>>17-(B*(D-ACIN)+P+CARRYIN)
      | P>>17-(B*A)
      | P>>17-(B*A+C)
      | P>>17-(B*A+C+CARRYIN)
      | P>>17-(B*A+CARRYIN)
      | P>>17-(B*A+P)
      | P>>17-(B*A+P+CARRYIN)
      | P>>17-(B*ACIN)
      | P>>17-(B*ACIN+C)
      | P>>17-(B*ACIN+C+CARRYIN)
      | P>>17-(B*ACIN+CARRYIN)
      | P>>17-(B*ACIN+P)
      | P>>17-(B*ACIN+P+CARRYIN)
      | P>>17-(B*D)
      | P>>17-(B*D+C)
      | P>>17-(B*D+C+CARRYIN)
      | P>>17-(B*D+CARRYIN)
      | P>>17-(B*D+P)
      | P>>17-(B*D+P+CARRYIN)
      | P>>17-(BCIN*(A+D))
      | P>>17-(BCIN*(A+D)+C)
      | P>>17-(BCIN*(A+D)+C+CARRYIN)
      | P>>17-(BCIN*(A+D)+CARRYIN)
      | P>>17-(BCIN*(A+D)+P)
      | P>>17-(BCIN*(A+D)+P+CARRYIN)
      | P>>17-(BCIN*(ACIN+D))
      | P>>17-(BCIN*(ACIN+D)+C)
      | P>>17-(BCIN*(ACIN+D)+C+CARRYIN)
      | P>>17-(BCIN*(ACIN+D)+CARRYIN)
      | P>>17-(BCIN*(ACIN+D)+P)
      | P>>17-(BCIN*(ACIN+D)+P+CARRYIN)
      | P>>17-(BCIN*(D+A))
      | P>>17-(BCIN*(D+A)+C)
      | P>>17-(BCIN*(D+A)+C+CARRYIN)
      | P>>17-(BCIN*(D+A)+CARRYIN)
      | P>>17-(BCIN*(D+A)+P)
      | P>>17-(BCIN*(D+A)+P+CARRYIN)
      | P>>17-(BCIN*(D+ACIN))